simulink model "non_linear_final"  (R2021b)
settings: Solver=ode23  StartTime=0.0  StopTime=30  RelTol=1e-3
values from neighbouring files (symbols this model uses):
  A_ratio = 1.41    [non_linear_param_final.m]
  Annulus_area = Bore_area-Rod_area    (= 2356.19449019)    [non_linear_param_final.m]
  beta_F = 12000e5    (= 1200000000)    [non_linear_param_final.m]
  Bore_area = pi/4*Bore_diameter^2    (= 3318.30724035)    [non_linear_param_final.m]
  cyl_c_end = 9e8    (= 900000000)    [non_linear_param_final.m]
  cyl_c_visc = 1500    [non_linear_param_final.m]
  cyl_CH_stat = 1/50    (= 0.02)    [non_linear_param_final.m]
  cyl_F_coul = 75    [non_linear_param_final.m]
  cyl_FH_stat = 500    [non_linear_param_final.m]
  cyl_gamma = 250    [non_linear_param_final.m]
  cyl_k_end = 6e9    (= 6000000000)    [non_linear_param_final.m]
  DB = -0.114    [non_linear_param_final.m]
  dr_dcv = 0.7    [non_linear_param_final.m]
  Eff_Bulk_On = 1    [non_linear_param_final.m]
  eta_air = 0.7e-2    (= 0.007)    [non_linear_param_final.m]
  k_cv = 15    [non_linear_param_final.m]
  k_DCV_PA = 7.9    [non_linear_param_final.m]
  k_DCV_PB = 7.78    [non_linear_param_final.m]
  k_DCV_T = 7.9    [non_linear_param_final.m]
  k_ocv = 15    [non_linear_param_final.m]
  K_oil = 1.5e4*0+12000    (= 12000)    [non_linear_param_final.m]
  k_tanh = 0.005    [non_linear_param_final.m]
  kappa_air = 1.4    [non_linear_param_final.m]
  LargeValveOpening = 1    [non_linear_param_final.m]
  mu_ocv = 3    [non_linear_param_final.m]
  OL_pa = -0.114    [non_linear_param_final.m]
  OL_pb = 0.114    [non_linear_param_final.m]
  p0_A = 62.79    [non_linear_param_final.m]
  p0_A1 = 1.08    [non_linear_param_final.m]
  p0_B = 1.213    [non_linear_param_final.m]
  p_cv_fo = 1    [non_linear_param_final.m]
  p_cv_set = 2.8    [non_linear_param_final.m]
  p_ocv_fo = 350    [non_linear_param_final.m]
  p_ocv_set = 150    [non_linear_param_final.m]
  p_pc_set = 7    [non_linear_param_final.m]
  p_R = 1.1    [non_linear_param_final.m]
  p_S = 180    [non_linear_param_final.m]
  patm = 1.013e5    (= 101300)    [non_linear_param_final.m]
  Stroke = 500    [non_linear_param_final.m]
  t0 = 1    [non_linear_param_final.m]
  tau_ocv = 0.0889*0+0.055    (= 0.055)    [non_linear_param_final.m]
  tau_pc = 0.0064*0+0.009    (= 0.009)    [non_linear_param_final.m]
  tStepDown = 4.7*0+4.6    (= 4.6)    [non_linear_param_final.m]
  tStepUp = 6.2    [non_linear_param_final.m]
  tWait = 8.0    (= 8)    [non_linear_param_final.m]
  UL_at = -0.05    [non_linear_param_final.m]
  UL_bt = 0.05    [non_linear_param_final.m]
  Up = 1    [non_linear_param_final.m]
  uS_sp = -0.55    [non_linear_param_final.m]
  V_CH_A = 1.0e-03    (= 0.001)    [non_linear_param_final.m]
  V_CH_A1 = 0.1e-03    (= 0.0001)    [non_linear_param_final.m]
  V_CH_B = 1.25e-03    (= 0.00125)    [non_linear_param_final.m]
  v_cyl_0 = 0    [non_linear_param_final.m]
  wn_dcv = 30    [non_linear_param_final.m]
  x_cyl_0 = 0.45012    [non_linear_param_final.m]
undefined symbols (not defined in the file or in its folder): sigbuilder_block, uS0
libraries: eeGeneralControl x3
(part 2 of 2)

== system non_linear_final/Directional Control Valve/Valve Dynamics ==
  note: 2nd order TF for
      | DCV dynamics
  u_dcv_ref [-] : Inport
  2nd order TF : TransferFcn  Denominator="[1/(wn_dcv^2) 2*dr_dcv/wn_dcv  1]"
  u_dcv [-] : Outport  VectorParamsAs1DForOutWhenUnconnected=off
  tag scope: u_dcv=global
  wires:
  u_dcv_ref [-]/1 -> 2nd order TF/1  "u_dcv_ref [-]"
  2nd order TF/1 -> [u_dcv], u_dcv [-]/1  "u_dcv [-]"

== system non_linear_final/Monitoring/Subsystem ==
  Constant1 : Constant  Value=A_ratio
  Divide3 : Product  Inputs=**
  Gain15 : Gain  Gain=-1
  Q_A exp [L/min] : Outport  VectorParamsAs1DForOutWhenUnconnected=off
  wires:
  [Q_B_exp] -> Divide3/1
  Gain15/1 -> Q_A exp [L/min]/1  "Q_A exp [L/min]"
  Constant1/1 -> Divide3/2
  Divide3/1 -> Gain15/1

== system non_linear_final/Over-Centre Valve/Capasitance ==
  Bulk Modulus : Subsystem
  Constant : Constant  Value=V_CH_A1
  Divide : Product  Inputs=*/
  "CH_A1 " : Outport  VectorParamsAs1DForOutWhenUnconnected=off
  wires:
  Bulk Modulus/1(K_oil) -> Divide/2  "[bar]"
  Constant/1 -> Divide/1  "[m^3]"
  Divide/1 -> "CH_A1 "/1

== system non_linear_final/Over-Centre Valve/Check Valve ==
  p_A [bar] : Inport
  p_A1 [bar] : Inport  Port=2
  Abs : Abs  ZeroCross=off
  Constant : Constant  Value=k_cv
  Product : Product  Inputs=****
  Saturation : Saturate  UpperLimit=1  LowerLimit=0  ZeroCross=off
  Sign : Subsystem
  Sqrt : Sqrt  SaturateOnIntegerOverflow=on
  Sum : Sum  Inputs=+-
  Sum1 : Sum  Inputs=+-
  division by p_cv_fo : Gain  Gain=1/p_cv_fo
  p_cv_set [bar] : Constant  Value=p_cv_set
  Q_cv [l/min]1 : Outport  VectorParamsAs1DForOutWhenUnconnected=off
  tag scope: uCV=global
  wires:
  p_cv_set [bar]/1 -> Sum1/2
  Sum/1 -> Abs/1, Sum1/1, Sign/1(In)  "p2-p3 [bar]"
  p_A [bar]/1 -> Sum/2
  p_A1 [bar]/1 -> Sum/1
  division by p_cv_fo/1 -> Saturation/1  "u_cv [-]"
  Sum1/1 -> division by p_cv_fo/1
  Constant/1 -> Product/1  "[l/min/bar^0.5]"
  Sqrt/1 -> Product/2  "[bar^0.5]"
  Sign/1(Out) -> Product/4
  Saturation/1 -> [uCV], Product/3  "[-]"
  Abs/1 -> Sqrt/1
  Product/1 -> Q_cv [l/min]1/1

== system non_linear_final/Over-Centre Valve/Over-Centre Valve ==
  u_ocv [-] : Inport
  p_A [bar] : Inport  Port=2
  p_A1 [bar] : Inport  Port=3
  Abs : Abs  ZeroCross=off
  Product : Product  Inputs=****
  Sign : Subsystem
  Sqrt : Sqrt  SaturateOnIntegerOverflow=on
  k_ocv [l/min/bar^0.5] : Constant  Value=k_ocv
  p3-p2 : Sum  Inputs=+-
  "[l/min]" : Outport  VectorParamsAs1DForOutWhenUnconnected=off
  wires:
  k_ocv [l/min/bar^0.5]/1 -> Product/3
  p3-p2/1 -> Sign/1(In), Abs/1  "p3-p2 [bar]"
  Sqrt/1 -> Product/2  "[bar^0.5]"
  Sign/1(Out) -> Product/4
  p_A1 [bar]/1 -> p3-p2/2
  p_A [bar]/1 -> p3-p2/1
  Abs/1 -> Sqrt/1
  u_ocv [-]/1 -> Product/1  "[-]"
  Product/1 -> "[l/min]"/1

== system non_linear_final/Over-Centre Valve/Pressure Equillibrium ==
  p_B [bar] : Inport
  p_A [bar] : Inport  Port=2
  Division by p_ocv_fo [bar] : Gain  Gain=1/p_ocv_fo
  Pilot ratio [-] : Gain  Gain=mu_ocv
  Saturation : Saturate  UpperLimit=1  LowerLimit=0  ZeroCross=off
  Sum : Sum  Inputs=++-
  Valve Dynamics : TransferFcn  Denominator=[tau_ocv 1]
  p_ocv_set [bar] : Constant  Value=p_ocv_set
  u_ocv [-] : Outport  VectorParamsAs1DForOutWhenUnconnected=off
  tag scope: uOCV=global
  wires:
  Sum/1 -> Division by p_ocv_fo [bar]/1  "[bar]"
  Pilot ratio [-]/1 -> Sum/1
  p_ocv_set [bar]/1 -> Sum/3
  p_B [bar]/1 -> Pilot ratio [-]/1
  p_A [bar]/1 -> Sum/2
  Valve Dynamics/1 -> [uOCV], u_ocv [-]/1  "[-]"
  Division by p_ocv_fo [bar]/1 -> Saturation/1  "u_ocv_ref [-]"
  Saturation/1 -> Valve Dynamics/1

== system non_linear_final/Step Input Generator/VCC_Step_Function_Validation ==
  Enable : Inport  OutDataTypeStr=boolean
  u_SP : Inport  Port=2
  uS0 : Inport  Port=3
  DB : Inport  Port=4
  CyclicTime : Inport  Port=5
  t0 : Inport  Port=6
  tHold1 : Inport  Port=7
  tStep : Inport  Port=8
  tHold2 : Inport  Port=9
  Constant : Constant  Value=0
  StepFunction : MATLAB Function  TreatAsAtomicUnit=on
  Switch : Switch  Criteria=u2 > Threshold
  u_ref : Outport  VectorParamsAs1DForOutWhenUnconnected=off
  EndTime : Outport  Port=2  VectorParamsAs1DForOutWhenUnconnected=off
  wires:
  u_SP/1 -> StepFunction/1(u_SP)
  tStep/1 -> StepFunction/7(t2)
  tHold1/1 -> StepFunction/6(t1)
  t0/1 -> StepFunction/5(t0)
  CyclicTime/1 -> StepFunction/4(t)
  StepFunction/2(T) -> EndTime/1
  uS0/1 -> StepFunction/2(uS0)
  tHold2/1 -> StepFunction/8(t3)
  DB/1 -> StepFunction/3(DB)
  StepFunction/1(y) -> Switch/1
  Switch/1 -> u_ref/1
  Constant/1 -> Switch/3
  Enable/1 -> Switch/2

== system non_linear_final/Step Input Generator/VCC_Step_Function_Validation1 ==
  Enable : Inport  OutDataTypeStr=boolean
  u_SP : Inport  Port=2
  uS0 : Inport  Port=3
  DB : Inport  Port=4
  CyclicTime : Inport  Port=5
  t0 : Inport  Port=6
  tHold1 : Inport  Port=7
  tStep : Inport  Port=8
  tHold2 : Inport  Port=9
  Constant : Constant  Value=0
  StepFunction : MATLAB Function  TreatAsAtomicUnit=on
  Switch : Switch  Criteria=u2 > Threshold
  u_ref : Outport  VectorParamsAs1DForOutWhenUnconnected=off
  EndTime : Outport  Port=2  VectorParamsAs1DForOutWhenUnconnected=off
  wires:
  u_SP/1 -> StepFunction/1(u_SP)
  tStep/1 -> StepFunction/7(t2)
  tHold1/1 -> StepFunction/6(t1)
  t0/1 -> StepFunction/5(t0)
  CyclicTime/1 -> StepFunction/4(t)
  StepFunction/2(T) -> EndTime/1
  uS0/1 -> StepFunction/2(uS0)
  tHold2/1 -> StepFunction/8(t3)
  DB/1 -> StepFunction/3(DB)
  StepFunction/1(y) -> Switch/1
  Switch/1 -> u_ref/1
  Constant/1 -> Switch/3
  Enable/1 -> Switch/2

== system non_linear_final/Subsystem/CH Bore Side ==
  x [m] : Inport
  Bulk Modulus : Subsystem
  Constant4 : Constant  Value=V_CH_A
  Divide2 : Product  Inputs=*/
  Gain1 : Gain  Gain=Bore_area
  Gain2 : Gain  Gain=1/(10)^9
  Gain3 : Gain  Gain=1000
  Scope : Scope
  Sum3 : Sum
  CH_A : Outport  VectorParamsAs1DForOutWhenUnconnected=off
  wires:
  Sum3/1 -> Divide2/1  "[m^3]"
  x [m]/1 -> Gain3/1
  Constant4/1 -> Sum3/1  "[m^3]"
  Gain1/1 -> Gain2/1  "[mm^3]"
  Gain3/1 -> Gain1/1  "[mm]"
  Gain2/1 -> Scope/1, Sum3/2  "[m^3]"
  Divide2/1 -> CH_A/1
  Bulk Modulus/1(K_oil) -> Divide2/2  "[bar]"

== system non_linear_final/Subsystem/CH Rod Side ==
  x [m] : Inport
  Bulk Modulus : Subsystem
  Constant1 : Constant  Value=Stroke
  Constant4 : Constant  Value=V_CH_B
  Divide2 : Product  Inputs=*/
  Gain1 : Gain  Gain=Annulus_area
  Gain2 : Gain  Gain=1/(10)^9
  Gain4 : Gain  Gain=1000
  Scope : Scope
  Sum2 : Sum  Inputs=+-
  Sum3 : Sum
  CH_B : Outport  VectorParamsAs1DForOutWhenUnconnected=off
  wires:
  Sum3/1 -> Divide2/1  "[m^3]"
  Constant1/1 -> Sum2/1  "[mm]"
  x [m]/1 -> Gain4/1
  Sum2/1 -> Gain1/1
  Constant4/1 -> Sum3/1  "[m^3]"
  Gain1/1 -> Gain2/1  "[mm^3]"
  Gain4/1 -> Sum2/2  "[mm]"
  Gain2/1 -> Sum3/2, Scope/1  "[m^3]"
  Divide2/1 -> CH_B/1
  Bulk Modulus/1(K_oil) -> Divide2/2

== system non_linear_final/Subsystem/Cylinder forces ==
  v_cyl [m/s] : Inport
  F_hyd [N] : Inport  Port=2
  x _cyl [m] : Inport  Port=3
  Add : Sum  Inputs=+-+-
  Lower Stroke Limit : Subsystem
  Stribeck Friction1 : Subsystem
  Upper Stroke Limit : Subsystem
  F_piston [N] : Outport  VectorParamsAs1DForOutWhenUnconnected=off
  wires:
  Lower Stroke Limit/1(F_cyl_contact [N]) -> Add/3
  Upper Stroke Limit/1(F_cyl_contact [N]) -> Add/2
  x _cyl [m]/1 -> Upper Stroke Limit/1(x_cyl [m]), Lower Stroke Limit/1(x_cyl [m])
  Add/1 -> F_piston [N]/1  "Bm_Cyl_F"
  v_cyl [m/s]/1 -> Stribeck Friction1/1(v_cyl [m/s]), Lower Stroke Limit/2(v_cyl [m]), Upper Stroke Limit/2(v_cyl [m/s])
  Stribeck Friction1/1(F [N]) -> Add/4
  F_hyd [N]/1 -> Add/1

== system non_linear_final/Subsystem1/VCC_Step_Function_Validation ==
  Enable : Inport  OutDataTypeStr=boolean
  u_SP : Inport  Port=2
  uS0 : Inport  Port=3
  DB : Inport  Port=4
  CyclicTime : Inport  Port=5
  t0 : Inport  Port=6
  tHold1 : Inport  Port=7
  tStep : Inport  Port=8
  tHold2 : Inport  Port=9
  Constant : Constant  Value=0
  StepFunction : MATLAB Function  TreatAsAtomicUnit=on
  Switch : Switch  Criteria=u2 > Threshold
  u_ref : Outport  VectorParamsAs1DForOutWhenUnconnected=off
  EndTime : Outport  Port=2  VectorParamsAs1DForOutWhenUnconnected=off
  wires:
  u_SP/1 -> StepFunction/1(u_SP)
  tStep/1 -> StepFunction/7(t2)
  tHold1/1 -> StepFunction/6(t1)
  t0/1 -> StepFunction/5(t0)
  CyclicTime/1 -> StepFunction/4(t)
  StepFunction/2(T) -> EndTime/1
  uS0/1 -> StepFunction/2(uS0)
  tHold2/1 -> StepFunction/8(t3)
  DB/1 -> StepFunction/3(DB)
  StepFunction/1(y) -> Switch/1
  Switch/1 -> u_ref/1
  Constant/1 -> Switch/3
  Enable/1 -> Switch/2

== system non_linear_final/Directional Control Valve/Edge Flows/Flow Characteristics ==
  u_B-T [-] : Inport
  p_B - p_R [bar] : Inport  Port=2
  Abs : Abs
  Constant : Constant  Value=k_DCV_T
  Product6 : Product  Inputs=4  RndMeth=Zero
  Sign : Subsystem
  Sqrt7 : Sqrt  SaturateOnIntegerOverflow=on
  Q_B-T [L/min] : Outport  VectorParamsAs1DForOutWhenUnconnected=off
  wires:
  Sqrt7/1 -> Product6/3  "[bar]^0.5"
  Product6/1 -> Q_B-T [L/min]/1  "[L/min]"
  p_B - p_R [bar]/1 -> Sign/1(In), Abs/1  "[bar]"
  Abs/1 -> Sqrt7/1
  u_B-T [-]/1 -> Product6/2  "[-]"
  Constant/1 -> Product6/1  "[L/min*bar^0.5]"
  Sign/1(Out) -> Product6/4

== system non_linear_final/Directional Control Valve/Edge Flows/Flow Characteristics1 ==
  u_A-T [-] : Inport
  p_A1 - p_R [bar] : Inport  Port=2
  Abs : Abs
  Constant : Constant  Value=k_DCV_T
  Product6 : Product  Inputs=4  RndMeth=Zero
  Sign : Subsystem
  Sqrt7 : Sqrt  SaturateOnIntegerOverflow=on
  Q_A-T [L/min] : Outport  VectorParamsAs1DForOutWhenUnconnected=off
  wires:
  Sqrt7/1 -> Product6/3  "[bar]^0.5"
  Product6/1 -> Q_A-T [L/min]/1  "[L/min]"
  p_A1 - p_R [bar]/1 -> Sign/1(In), Abs/1  "[bar]"
  Abs/1 -> Sqrt7/1
  u_A-T [-]/1 -> Product6/2  "[-]"
  Sign/1(Out) -> Product6/4
  Constant/1 -> Product6/1  "[L/min*bar^0.5]"

== system non_linear_final/Directional Control Valve/Edge Flows/Flow Characteristics2 ==
  u_P-A [-] : Inport
  p_C - p_A1 [bar] : Inport  Port=2
  Abs : Abs
  Constant : Constant  Value=k_DCV_PA
  Product6 : Product  Inputs=4  RndMeth=Zero
  Sign : Subsystem
  Sqrt7 : Sqrt  SaturateOnIntegerOverflow=on
  Q_P-A [L/min] : Outport  VectorParamsAs1DForOutWhenUnconnected=off
  wires:
  Sqrt7/1 -> Product6/3  "[bar]^0.5"
  Product6/1 -> Q_P-A [L/min]/1  "[L/min]"
  p_C - p_A1 [bar]/1 -> Sign/1(In), Abs/1  "[bar]"
  Abs/1 -> Sqrt7/1
  u_P-A [-]/1 -> Product6/2  "[-]"
  Sign/1(Out) -> Product6/4
  Constant/1 -> Product6/1  "[L/min*bar^0.5]"

== system non_linear_final/Directional Control Valve/Edge Flows/Flow Characteristics3 ==
  u_P-B [-] : Inport
  p_C - p_B [bar] : Inport  Port=2
  Abs : Abs
  Constant : Constant  Value=k_DCV_PB
  Product6 : Product  Inputs=4  RndMeth=Zero
  Sign : Subsystem
  Sqrt7 : Sqrt  SaturateOnIntegerOverflow=on
  Q_P-B [L/min] : Outport  VectorParamsAs1DForOutWhenUnconnected=off
  wires:
  Sqrt7/1 -> Product6/3  "[bar]^0.5"
  Product6/1 -> Q_P-B [L/min]/1  "[L/min]"
  p_C - p_B [bar]/1 -> Sign/1(In), Abs/1  "[bar]"
  Abs/1 -> Sqrt7/1
  u_P-B [-]/1 -> Product6/2  "[-]"
  Sign/1(Out) -> Product6/4
  Constant/1 -> Product6/1  "[L/min*bar^0.5]"

== system non_linear_final/Directional Control Valve/Edge Flows/Q_2 selection ==
  Q_P-A [l/min] : Inport
  Q_P-B [l/min] : Inport  Port=2
  Sum : Sum
  Q_DCV_In [l/min] : Outport  VectorParamsAs1DForOutWhenUnconnected=off
  wires:
  Sum/1 -> Q_DCV_In [l/min]/1
  Q_P-B [l/min]/1 -> Sum/2
  Q_P-A [l/min]/1 -> Sum/1

== system non_linear_final/Directional Control Valve/Edge Openings/Edge A-T ==
  u_dcv [-] : Inport
  MATLAB Function1 : MATLAB Function  TreatAsAtomicUnit=on
  u_A-T [-] : Outport  VectorParamsAs1DForOutWhenUnconnected=off
  wires:
  MATLAB Function1/1(u_AT) -> u_A-T [-]/1
  u_dcv [-]/1 -> MATLAB Function1/1(u_dcv)

== system non_linear_final/Directional Control Valve/Edge Openings/Edge B-T ==
  u_dcv [-] : Inport
  MATLAB Function : MATLAB Function  TreatAsAtomicUnit=on
  u_B-T [-] : Outport  VectorParamsAs1DForOutWhenUnconnected=off
  wires:
  u_dcv [-]/1 -> MATLAB Function/1(u_dcv)
  MATLAB Function/1(u_BT) -> u_B-T [-]/1

== system non_linear_final/Directional Control Valve/Edge Openings/Edge P-A ==
  u_dcv [-] : Inport
  MATLAB Function1 : MATLAB Function  TreatAsAtomicUnit=on
  u_P-A [-] : Outport  VectorParamsAs1DForOutWhenUnconnected=off
  wires:
  u_dcv [-]/1 -> MATLAB Function1/1(u_dcv)
  MATLAB Function1/1(u_PA) -> u_P-A [-]/1

== system non_linear_final/Directional Control Valve/Edge Openings/Edge P-B ==
  u_dcv [-] : Inport
  MATLAB Function1 : MATLAB Function  TreatAsAtomicUnit=on
  u_P-B [-] : Outport  VectorParamsAs1DForOutWhenUnconnected=off
  wires:
  u_dcv [-]/1 -> MATLAB Function1/1(u_dcv)
  MATLAB Function1/1(u_PB) -> u_P-B [-]/1

== system non_linear_final/Over-Centre Valve/Capasitance/Bulk Modulus ==
  Bulk/Volume : Constant  Value=K_oil
  Eff_Bulk_On : Constant  Value=Eff_Bulk_On
  Effective Bulk Modulus : Subsystem
  Switch : Switch  Criteria=u2 > Threshold
  K_oil : Outport  VectorParamsAs1DForOutWhenUnconnected=off
  wires:
  Bulk/Volume/1 -> Switch/3  "[bar]"
  Switch/1 -> K_oil/1
  Eff_Bulk_On/1 -> Switch/2
  Effective Bulk Modulus/1(K_oil_eff) -> Switch/1  "[bar]"

== system non_linear_final/Over-Centre Valve/Check Valve/Sign ==
  In : Inport
  Gain2 : Gain  Gain=1/k_tanh
  Trigonometric Function : Trigonometry  Operator=tanh
  Out : Outport  VectorParamsAs1DForOutWhenUnconnected=off
  wires:
  In/1 -> Gain2/1
  Gain2/1 -> Trigonometric Function/1
  Trigonometric Function/1 -> Out/1  "[-]"

== system non_linear_final/Over-Centre Valve/Over-Centre Valve/Sign ==
  In : Inport
  Gain2 : Gain  Gain=1/k_tanh
  Trigonometric Function : Trigonometry  Operator=tanh
  Out : Outport  VectorParamsAs1DForOutWhenUnconnected=off
  wires:
  In/1 -> Gain2/1
  Gain2/1 -> Trigonometric Function/1
  Trigonometric Function/1 -> Out/1  "[-]"

== system non_linear_final/Subsystem/CH Bore Side/Bulk Modulus ==
  Bulk/Volume : Constant  Value=K_oil
  Eff_Bulk_On : Constant  Value=Eff_Bulk_On
  Effective Bulk Modulus : Subsystem
  Switch : Switch  Criteria=u2 > Threshold
  K_oil : Outport  VectorParamsAs1DForOutWhenUnconnected=off
  wires:
  Bulk/Volume/1 -> Switch/3  "[bar]"
  Switch/1 -> K_oil/1
  Eff_Bulk_On/1 -> Switch/2
  Effective Bulk Modulus/1(K_oil_eff) -> Switch/1  "[bar]"

== system non_linear_final/Subsystem/CH Rod Side/Bulk Modulus ==
  Bulk/Volume : Constant  Value=K_oil
  Eff_Bulk_On : Constant  Value=Eff_Bulk_On
  Effective Bulk Modulus : Subsystem
  Switch : Switch  Criteria=u2 > Threshold
  K_oil : Outport  VectorParamsAs1DForOutWhenUnconnected=off
  wires:
  Bulk/Volume/1 -> Switch/3  "[bar]"
  Switch/1 -> K_oil/1
  Eff_Bulk_On/1 -> Switch/2
  Effective Bulk Modulus/1(K_oil_eff) -> Switch/1  "[bar]"

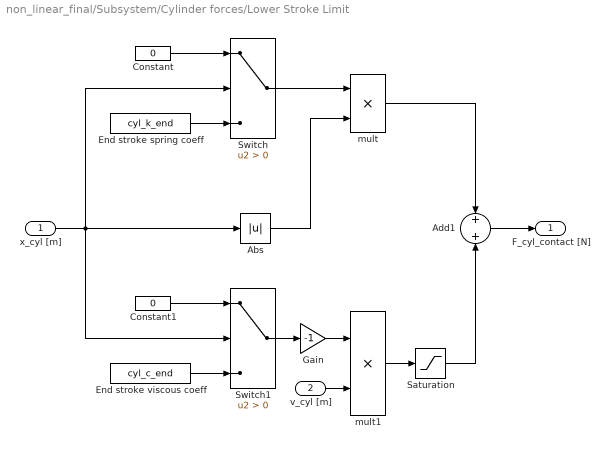
== system non_linear_final/Subsystem/Cylinder forces/Lower Stroke Limit ==
  x_cyl [m] : Inport
  v_cyl [m] : Inport  Port=2
  Abs : Abs
  Add1 : Sum
  Constant : Constant  Value=0
  Constant1 : Constant  Value=0
  End stroke spring coeff : Constant  Value=cyl_k_end
  End stroke viscous coeff : Constant  Value=cyl_c_end
  Gain : Gain  Gain=-1
  Saturation : Saturate  UpperLimit=0  LowerLimit=-inf
  Switch : Switch  Criteria=u2 > Threshold
  Switch1 : Switch  Criteria=u2 > Threshold
  mult : Product  Inputs=**
  mult1 : Product  Inputs=**
  F_cyl_contact [N] : Outport  VectorParamsAs1DForOutWhenUnconnected=off
  wires:
  Add1/1 -> F_cyl_contact [N]/1
  mult1/1 -> Saturation/1
  mult/1 -> Add1/1
  Switch1/1 -> Gain/1
  Abs/1 -> mult/2
  Switch/1 -> mult/1
  x_cyl [m]/1 -> Switch1/2, Abs/1, Switch/2
  Constant/1 -> Switch/1
  End stroke spring coeff/1 -> Switch/3
  End stroke viscous coeff/1 -> Switch1/3
  Constant1/1 -> Switch1/1
  Gain/1 -> mult1/1
  v_cyl [m]/1 -> mult1/2
  Saturation/1 -> Add1/2

== system non_linear_final/Subsystem/Cylinder forces/Stribeck Friction1 ==
  v_cyl [m/s] : Inport
  Mux : Mux  Inputs=6
  Stribeck Curve : Fcn  Expr=u(1)*u(2)+tanh(u(1)*u(3))*(u(4)+u(5)*exp(-(u(1)*tanh(u(1)*u(3)))/u(6)))
  "coefficient of viscous friction " : Constant  Value=cyl_c_visc
  coulomb friction : Constant  Value=cyl_F_coul
  friction force approximation constant : Constant  Value=cyl_gamma
  friction time constant : Constant  Value=cyl_CH_stat
  static friction : Constant  Value=cyl_FH_stat
  F [N] : Outport  VectorParamsAs1DForOutWhenUnconnected=off
  wires:
  v_cyl [m/s]/1 -> Mux/1
  Stribeck Curve/1 -> F [N]/1
  friction time constant/1 -> Mux/6
  static friction/1 -> Mux/5
  coulomb friction/1 -> Mux/4
  friction force approximation constant/1 -> Mux/3
  "coefficient of viscous friction "/1 -> Mux/2
  Mux/1 -> Stribeck Curve/1

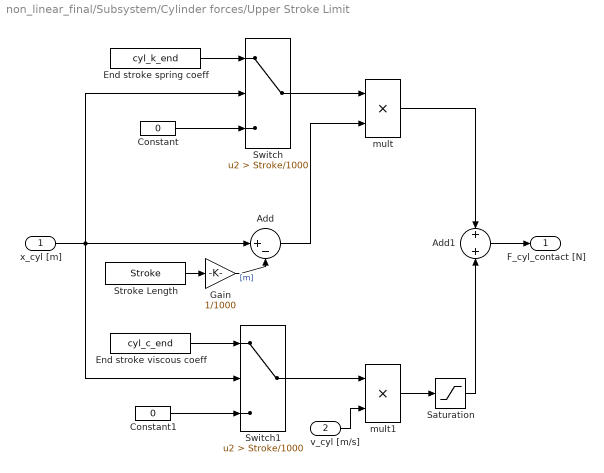
== system non_linear_final/Subsystem/Cylinder forces/Upper Stroke Limit ==
  x_cyl [m] : Inport
  v_cyl [m/s] : Inport  Port=2
  Add : Sum  Inputs=|+-
  Add1 : Sum
  Constant : Constant  Value=0
  Constant1 : Constant  Value=0
  End stroke spring coeff : Constant  Value=cyl_k_end
  End stroke viscous coeff : Constant  Value=cyl_c_end
  Gain : Gain  Gain=1/1000
  Saturation : Saturate  UpperLimit=inf  LowerLimit=0
  Stroke Length : Constant  Value=Stroke
  Switch : Switch  Criteria=u2 > Threshold  Threshold=Stroke/1000
  Switch1 : Switch  Criteria=u2 > Threshold  Threshold=Stroke/1000
  mult : Product  Inputs=**
  mult1 : Product  Inputs=**
  F_cyl_contact [N] : Outport  VectorParamsAs1DForOutWhenUnconnected=off
  wires:
  x_cyl [m]/1 -> Add/1, Switch1/2, Switch/2
  End stroke spring coeff/1 -> Switch/1
  Constant/1 -> Switch/3
  Stroke Length/1 -> Gain/1
  Switch/1 -> mult/1
  Add/1 -> mult/2
  End stroke viscous coeff/1 -> Switch1/1
  Constant1/1 -> Switch1/3
  Switch1/1 -> mult1/1
  mult/1 -> Add1/1
  mult1/1 -> Saturation/1
  Add1/1 -> F_cyl_contact [N]/1
  v_cyl [m/s]/1 -> mult1/2
  Saturation/1 -> Add1/2
  Gain/1 -> Add/2  "[m]"

== system non_linear_final/Directional Control Valve/Edge Flows/Flow Characteristics/Sign ==
  In : Inport
  Gain2 : Gain  Gain=1/k_tanh
  Trigonometric Function : Trigonometry  Operator=tanh
  Out : Outport  VectorParamsAs1DForOutWhenUnconnected=off
  wires:
  In/1 -> Gain2/1
  Gain2/1 -> Trigonometric Function/1
  Trigonometric Function/1 -> Out/1  "[-]"

== system non_linear_final/Directional Control Valve/Edge Flows/Flow Characteristics1/Sign ==
  In : Inport
  Gain2 : Gain  Gain=1/k_tanh
  Trigonometric Function : Trigonometry  Operator=tanh
  Out : Outport  VectorParamsAs1DForOutWhenUnconnected=off
  wires:
  In/1 -> Gain2/1
  Gain2/1 -> Trigonometric Function/1
  Trigonometric Function/1 -> Out/1  "[-]"

== system non_linear_final/Directional Control Valve/Edge Flows/Flow Characteristics2/Sign ==
  In : Inport
  Gain2 : Gain  Gain=1/k_tanh
  Trigonometric Function : Trigonometry  Operator=tanh
  Out : Outport  VectorParamsAs1DForOutWhenUnconnected=off
  wires:
  In/1 -> Gain2/1
  Gain2/1 -> Trigonometric Function/1
  Trigonometric Function/1 -> Out/1  "[-]"

== system non_linear_final/Directional Control Valve/Edge Flows/Flow Characteristics3/Sign ==
  In : Inport
  Gain2 : Gain  Gain=1/k_tanh
  Trigonometric Function : Trigonometry  Operator=tanh
  Out : Outport  VectorParamsAs1DForOutWhenUnconnected=off
  wires:
  In/1 -> Gain2/1
  Gain2/1 -> Trigonometric Function/1
  Trigonometric Function/1 -> Out/1  "[-]"

== system non_linear_final/Over-Centre Valve/Capasitance/Bulk Modulus/Effective Bulk Modulus ==
  2 : Scope
  MATLAB Function : MATLAB Function  TreatAsAtomicUnit=on
  K_oil_eff : Outport  VectorParamsAs1DForOutWhenUnconnected=off
  wires:
  MATLAB Function/1(K_oil_eff) -> K_oil_eff/1, 2/1
  [p_A1] -> MATLAB Function/1(p)

== system non_linear_final/Subsystem/CH Bore Side/Bulk Modulus/Effective Bulk Modulus ==
  MATLAB Function : MATLAB Function  TreatAsAtomicUnit=on
  K_oil_eff : Outport  VectorParamsAs1DForOutWhenUnconnected=off
  wires:
  MATLAB Function/1(K_oil_eff) -> K_oil_eff/1
  [p_A] -> MATLAB Function/1(p)

== system non_linear_final/Subsystem/CH Rod Side/Bulk Modulus/Effective Bulk Modulus ==
  MATLAB Function : MATLAB Function  TreatAsAtomicUnit=on
  K_oil_eff : Outport  VectorParamsAs1DForOutWhenUnconnected=off
  wires:
  MATLAB Function/1(K_oil_eff) -> K_oil_eff/1
  [p_B] -> MATLAB Function/1(p)

== matlab function non_linear_final/Directional Control Valve/Edge Openings/Edge A-T/MATLAB Function1 ==
  data: u_dcv input; u_AT output; OL_pa parameter; OL_pb parameter; UL_at parameter; UL_bt parameter
function u_AT = fcn(u_dcv,OL_pa, OL_pb, UL_at, UL_bt)

u_AT = 0;

if u_dcv <= UL_at
 u_AT = 0;
 
elseif u_dcv > UL_at   && u_dcv < 2*OL_pb
 u_AT= ((u_dcv-UL_at)*OL_pb)/((1-OL_pb)*(-UL_at+2*OL_pb));
 
elseif 2*OL_pb <= u_dcv
  u_AT= (u_dcv-OL_pb)/(1-OL_pb);
end

== matlab function non_linear_final/Subsystem1/VCC_Step_Function_Validation/StepFunction ==
  data: u_SP input; uS0 input; DB input; t input; t0 input; t1 input; t2 input; y output; T output; t3 input
function [y, T] = step(u_SP, uS0, DB,  t, t0,t1,t2,t3)
y = 0;



if t>=0 && t<t0
    y = uS0;
elseif t>=(t0) && t<(t0+t1)
    y = u_SP+DB; 
elseif t>=(t0+t1) && t<(t0+t1+t2)
    y = uS0; 
elseif t>=(t0+t1+t2) && t<(t0+t1+t2+t3)
    y = -u_SP-DB;          
else
    y = 0; 
end


T = t0+t1+t2+t3;

== matlab function non_linear_final/Directional Control Valve/Edge Openings/Edge B-T/MATLAB Function ==
  data: u_dcv input; u_BT output; OL_pa parameter; OL_pb parameter; UL_at parameter; UL_bt parameter
function u_BT = fcn(u_dcv,OL_pa, OL_pb, UL_at, UL_bt)

  u_BT = 0;

if UL_bt <=  u_dcv 
 u_BT = 0;
 
elseif 2*OL_pa < u_dcv && u_dcv < UL_bt
 u_BT= ((u_dcv-UL_bt)*OL_pa)/((1+OL_pa)*(UL_bt-2*OL_pa));
 
elseif u_dcv <=  2*OL_pa
  u_BT= -(u_dcv-OL_pa)/(1+OL_pa);
end

== matlab function non_linear_final/Step Input Generator/VCC_Step_Function_Validation/StepFunction ==
  data: u_SP input; uS0 input; DB input; t input; t0 input; t1 input; t2 input; y output; T output; t3 input
function [y, T] = step(u_SP, uS0, DB,  t, t0,t1,t2,t3)
y = 0;



if t>=0 && t<t0
    y = uS0;
elseif t>=(t0) && t<(t0+t1)
    y = u_SP+DB; 
elseif t>=(t0+t1) && t<(t0+t1+t2)
    y = uS0; 
elseif t>=(t0+t1+t2) && t<(t0+t1+t2+t3)
    y = -u_SP-DB;          
else
    y = 0; 
end


T = t0+t1+t2+t3;

== matlab function non_linear_final/Step Input Generator/VCC_Step_Function_Validation1/StepFunction ==
  data: u_SP input; uS0 input; DB input; t input; t0 input; t1 input; t2 input; y output; T output; t3 input
function [y, T] = step(u_SP, uS0, DB,  t, t0,t1,t2,t3)
y = 0;



if t>=0 && t<t0
    y = uS0;
elseif t>=(t0) && t<(t0+t1)
    y = u_SP+DB; 
elseif t>=(t0+t1) && t<(t0+t1+t2)
    y = uS0; 
elseif t>=(t0+t1+t2) && t<(t0+t1+t2+t3)
    y = -u_SP-DB;          
else
    y = 0; 
end


T = t0+t1+t2+t3;

== matlab function non_linear_final/Pressure Compensator1/Compensator From Morten Bak Paper ==
  data: p0 input; p_C output; p_S input; p_LS input
function p_C = fcn(p0, p_S, p_LS)

p_C = p_LS;

if p0 <= (p_S-p_LS)
p_C = p_LS+p0;
elseif 0<(p_S-p_LS) < p0
p_C = p_S - p_LS; 
elseif (p_S-p_LS) <= 0
    p_C = p_LS;
end

== matlab function non_linear_final/Directional Control Valve/Edge Openings/Edge P-A/MATLAB Function1 ==
  data: u_dcv input; u_PA output; OL_pa parameter; OL_pb parameter; UL_at parameter; UL_bt parameter
function u_PA = fcn(u_dcv,OL_pa, OL_pb, UL_at, UL_bt)

  u_PA = 0;

if OL_pa <=  u_dcv 
 u_PA = 0;
 
elseif u_dcv <  OL_pa
  u_PA= -(u_dcv-OL_pa)/(1+OL_pa);
end

== matlab function non_linear_final/Directional Control Valve/Edge Openings/Edge P-B/MATLAB Function1 ==
  data: u_dcv input; u_PB output; OL_pa parameter; OL_pb parameter; UL_at parameter; UL_bt parameter
function u_PB = fcn(u_dcv,OL_pa, OL_pb, UL_at, UL_bt)

  u_PB = 0;

if u_dcv <=   OL_pb
 u_PB = 0;
 
elseif  OL_pb < u_dcv 
  u_PB = (u_dcv-OL_pb)/(1-OL_pb);
end

== matlab function non_linear_final/Over-Centre Valve/Capasitance/Bulk Modulus/Effective Bulk Modulus/MATLAB Function ==
  data: p input; K_oil_eff output; patm parameter; eta_air parameter; kappa_air parameter; beta_F parameter
function K_oil_eff = fcn(p,patm, eta_air, kappa_air, beta_F)
p = p*1e5;
% Effective bulk modulus 
beta_eff_Pa  = 1/(1/beta_F+1/(((1-eta_air)*(patm/(p+patm))^(-1/kappa_air)/eta_air+1)*kappa_air*(p+patm)));
K_oil_eff = beta_eff_Pa*1e-5;

== matlab function non_linear_final/Rigid Mechanical System - Green Crane Arm/Lasse Schmidt ==
  data: Geq output; Ceq output; Meq output; x input

function [Geq,Ceq, Meq]  = fcn(x)

% Mechanical Model Green Crane @ UiA Laboratory 

% Parameters 
L_AGx = 3139/1000;            % [m]
L_AGy = 64*(1/1000);          % [m]
L_ACx = 550*(1/1000);         % [m]
L_ACy = 130*(1/1000);         % [m]
L_ABx = 420*(1/1000);         % [m]
L_ABy = 1055*(1/1000);        % [m]
L_AB = sqrt(L_ABx^2+L_ABy^2); % [m]
L_AC = sqrt(L_ACx^2+L_ACy^2); % [m]
L_AG = sqrt(L_AGx^2+L_AGy^2); % [m]
m_cm = 402;                   % [kg]
J_A = 4251.2;                 % [kg*m^2]
J_cm = J_A-L_AG^2*m_cm;       % [kg*m^2]
L_min = 772*(1/1000);         % [m]
g = 9.82;                     % [m/s^2]

% Gravitational Load [N]
Geq = -0.2e1*sqrt(-(x-L_AB+L_min+L_AC)*(x+L_min-L_AC-L_AB)*(x+L_AB+L_min-L_AC)*(x+L_AB+L_min+L_AC))*g*m_cm*(cos(acos((L_AB^2+L_AC^2-(x+L_min)^2)/L_AC/L_AB/0.2e1)-atan(L_ABy/L_ABx)+atan(L_ACy/L_ACx)+atan(L_AGy/L_AGx))*L_AGx-sin(acos((L_AB^2+L_AC^2-(x+L_min)^2)/L_AC/L_AB/0.2e1)-atan(L_ABy/L_ABx)+atan(L_ACy/L_ACx)+atan(L_AGy/L_AGx))*L_AGy)*(x+L_min)/(x-L_AB+L_min+L_AC)/(x+L_min-L_AC-L_AB)/(x+L_AB+L_min-L_AC)/(x+L_AB+L_min+L_AC);

% Equalent Inertial Load [kg]
Meq = -4*(L_AGx^2*(x+L_min)*m_cm+L_AGy^2*(x+L_min)*m_cm+(x+L_min)*J_cm)*(x+L_min)/(-x+L_AB-L_min-L_AC)/(-x+L_AB-L_min+L_AC)/(x+L_AB+L_min+L_AC)/(x+L_AB+L_min-L_AC);

% Coriolis Force [N*(m/s)^2]
Ceq = 4*((L_AGx^2+L_AGy^2)*m_cm+J_cm)*(x+L_min)*(-L_AB^2+L_AC^2+(x+L_min)^2)*(L_AB^2-L_AC^2+(x+L_min)^2)/(x+L_min-L_AC-L_AB)^2/(x-L_AB+L_min+L_AC)^2/(x+L_AB+L_min+L_AC)^2/(x+L_AB+L_min-L_AC)^2;

== matlab function non_linear_final/Subsystem/CH Bore Side/Bulk Modulus/Effective Bulk Modulus/MATLAB Function ==
  data: p input; K_oil_eff output; patm parameter; eta_air parameter; kappa_air parameter; beta_F parameter
function K_oil_eff = fcn(p,patm, eta_air, kappa_air, beta_F)
p = p*1e5;
% Effective bulk modulus 
beta_eff_Pa  = 1/(1/beta_F+1/(((1-eta_air)*(patm/(p+patm))^(-1/kappa_air)/eta_air+1)*kappa_air*(p+patm)));
K_oil_eff = beta_eff_Pa*1e-5;

== matlab function non_linear_final/Subsystem/CH Rod Side/Bulk Modulus/Effective Bulk Modulus/MATLAB Function ==
  data: p input; K_oil_eff output; patm parameter; eta_air parameter; kappa_air parameter; beta_F parameter
function K_oil_eff = fcn(p,patm, eta_air, kappa_air, beta_F)
p = p*1e5;
% Effective bulk modulus 
beta_eff_Pa  = 1/(1/beta_F+1/(((1-eta_air)*(patm/(p+patm))^(-1/kappa_air)/eta_air+1)*kappa_air*(p+patm)));
K_oil_eff = beta_eff_Pa*1e-5;
